simulink model "zcu102_f_200msps_64i_4096c"  (R2021a)
settings: Solver=VariableStepDiscrete  StartTime=0.0  StopTime=10.0  RelTol=1e-3
undefined symbols (not defined in the file or in its folder): datetime, pfb_coeff_gen_calc, posixtime
libraries: xbsIndex_r4 x49646, casper_library_misc x229, casper_library_delays x133, xps_library x20, casper_library_sources x4, xbsReplacements_r4 x4
(part 1 of 502)
settings Model: ModifiedComment=casperlwa -- Wed Dec 18 16:21:52 2024 -- Version 5.0  SimulationMode=rapid-accelerator  Creator=mdexter  LastModifiedBy=casperlwa
settings Model: ModifiedHistory=|
  | casperlwa -- Wed Dec 18 16:21:52 2024 -- Version 5.0
  | 
  | kplant -- Fri Aug 11 09:07:04 2023 -- Version 1.663
  | Saving after compiling
  | 
  | kplant -- Thu Aug 10 22:12:33 2023 -- Version 1.662
  | Version 7.3.2.1 now has pipelines with a latency of 3 before the f-engine's ethernet block.
  | 
  | kplant -- Thu Aug 10 10:59:50 2023 -- Version 1.661
  | Version 7.3.2.0 increases the pipeline latency to 2 in front of the f-engine 40Gbe block and changes the target clock rate to 196 MHz.
  | 
  | kplant -- Wed Aug 09 13:06:15 2023 -- Version 1.660
  | Added pipeline blocks with a latency of 1 before each of the inputs to the 40Gb ethernet block that the f-engine uses.
  | 
  | kplant -- Tue Aug 08 14:00:49 2023 -- Version 1.659
  | 
  | kplant -- Tue Aug 08 13:55:20 2023 -- Version 1.658
  | Adding cosmic ray system version 6.0.1.0 which has the FIR and so far has only been compiled from snap2_f_200msps_64i_4096c_nopfb.slx
  | 
  | kplant -- Thu Apr 27 20:02:10 2023 -- Version 1.657
  | Saving after compile.
  | 
  | kplant -- Wed Apr 26 17:00:51 2023 -- Version 1.656
  | I removed the delay trigger option and changed it to an option to delay the input to the buffer, in order to be able to position the pulse in the center of the buffer.
  | 
  | kplant -- Fri Apr 07 12:31:25 2023 -- Version 1.655
  | Add cosmic ray version 5.0.0.1 and change main version to 7.3.0.2.
  | 
  | kplant -- Thu Apr 06 14:32:58 2023 -- Version 1.654
  | Saving after compiling 7.3.0.1.
  | 
  | jackh -- Thu Apr 06 12:53:56 2023 -- Version 1.653
  | 
  | Make CR block and mask block use sync_time, not telescope_time
  | 
  | kplant -- Fri Mar 24 06:24:14 2023 -- Version 1.652
  | Saving after compiling version 7.3 with cosmic ray system 5.0.0.
  | 
  | jackh -- Wed Mar 08 22:10:30 2023 -- Version 1.651
  | 
  | compiled
  | 
  | jackh -- Wed Mar 08 15:15:31 2023 -- Version 1.650
  | rev 7.2
  | 2 bits below EQ binary point
  | 
  | jackh -- Thu Feb 16 17:13:47 2023 -- Version 1.649
  | 
  | compiled
  | 
  | jackh -- Thu Feb 16 09:43:58 2023 -- Version 1.648
  | 
  | Remove hard-coded first 5-stages of shift
  | 
  | jackh -- Wed Feb 15 17:04:22 2023 -- Version 1.647
  | 
  | compiled
  | 
  | jackh -- Tue Feb 14 18:32:23 2023 -- Version 1.646
  | 
  | Change EQ fractional bits from 5 to 4
  | Rebuild FFT IP with all serial stages optional shift
  | 
  | jackh -- Thu Jan 05 10:26:17 2023 -- Version 1.645
  | v7.0.0 as compiled
  | 
  | jackh -- Wed Jan 04 19:36:26 2023 -- Version 1.644
  | 
  | jackh -- Wed Jan 04 19:34:58 2023 -- Version 1.643
  | v7.0.0.0
  | CR version 4.3.1
  | 
  | jackh -- Tue Dec 20 11:37:43 2022 -- Version 1.642
  | 
  | compiled 6.1. 4 x 0.003ns timing errors, only on unused CR 40GbE RX path.
  | 
  | 
  | jackh -- Fri Dec 16 15:06:04 2022 -- Version 1.641
  | 
  | jackh -- Fri Dec 16 14:46:34 2022 -- Version 1.640
  | 
  | jackh -- Fri Dec 16 14:13:03 2022 -- Version 1.639
  | 
  | jackh -- Fri Dec 16 13:41:06 2022 -- Version 1.638
  | 
  | 6.1 -- add per-signal flag threshold
  | 
  | jackh -- Fri Dec 16 11:47:24 2022 -- Version 1.637
  | 
  | jackh -- Fri Dec 16 11:47:00 2022 -- Version 1.636
  | 
  | jackh -- Thu Dec 15 20:54:27 2022 -- Version 1.635
  | 
  | Add acc_time registers
  | 
  | jackh -- Thu Dec 15 12:49:42 2022 -- Version 1.634
  | 
  | Include all 4 mask cores (whoops!)
  | 
  | jackh -- Thu Dec 15 12:39:59 2022 -- Version 1.633
  | 
  | Change max coarse delay to 4096 (previous compile didn't meet timing)
  | 
  | jackh -- Wed Dec 14 18:27:06 2022 -- Version 1.632
  | 
  | Add "mask" block, which allows zero-ing out spectra
  | whose total power exceed a runtime-controllable
  | threshold
  | 
  | kplant -- Wed Jun 01 13:17:05 2022 -- Version 1.631
  | 
  | kplant -- Wed Jun 01 07:18:09 2022 -- Version 1.630
  | 
  | kplant -- Wed Jun 01 06:41:48 2022 -- Version 1.629
  | Fixed telescope time input to cosmic ray block.
  | 
  | kplant -- Wed Jun 01 06:39:11 2022 -- Version 1.628
  | Commented out scopes
  | 
  | kplant -- Tue May 31 12:58:33 2022 -- Version 1.627
  | Cosmic ray version 4.1. Takes in telescope time, attempts to fix bug where trigger signal is blocked from starting packetizer.
  | 
  | kplant -- Tue May 31 12:56:22 2022 -- Version 1.626
  | 
  | kplant -- Tue May 31 09:44:09 2022 -- Version 1.625
  | 
  | jackh -- Fri May 13 14:05:09 2022 -- Version 1.624
  | 
  | Compiled 5.1.1.0 (CR rev 4)
  | 
  | jackh -- Thu May 12 12:01:29 2022 -- Version 1.623
  | 
  | Add PPS count register (which software already assumes exists!)
  | 
  | jackh -- Thu May 12 11:34:03 2022 -- Version 1.622
  | 
  | v5.1.0
  | Manual merge in of v4.0 CR module
  | 
  | jackh -- Thu Apr 14 04:49:04 2022 -- Version 1.621
  | 
  | jackh -- Wed Mar 23 12:35:09 2022 -- Version 1.620
  | 
  | Manually merce cosmic_ray_system.slx into the cosmic_ray subsystem
  | of this model. cosmic_ray_system appears to be version 3 (based on version register).
  | 
  | Bump main model version to 5.0.0.0
  | 
  | jackh -- Tue Jan 11 12:36:06 2022 -- Version 1.619
  | 
  | jackh -- Mon Jan 10 21:45:35 2022 -- Version 1.618
  | 
  | Bump bugfix
  | 
  | jackh -- Mon Jan 10 21:43:35 2022 -- Version 1.617
  | 
  | jackh -- Mon Jan 10 21:39:42 2022 -- Version 1.616
  | 
  | jackh -- Mon Jan 10 21:33:55 2022 -- Version 1.615
  | 
  | jackh -- Mon Jan 10 21:16:30 2022 -- Version 1.614
  | 
  | Fix bit width of sum(sq(ADC value)) -- was configured for 8 bit ADCs!
  | 
  | jackh -- Mon Nov 15 21:56:57 2021 -- Version 1.613
  | 
  | compiled
  | 
  | jackh -- Mon Nov 15 11:54:45 2021 -- Version 1.612
  | 
  | v4.6.0 -- add internal loopback for sync logic
  | 
  | jackh -- Fri Sep 17 19:23:29 2021 -- Version 1.611
  | 
  | Compiled with clean timing
  | 
  | 
  | jackh -- Fri Sep 17 13:13:59 2021 -- Version 1.610
  | 4.5.4 CR update
  | 
  | jackh -- Tue Sep 14 19:01:48 2021 -- Version 1.609
  | 
  | jackh -- Tue Sep 14 10:37:44 2021 -- Version 1.608
  | 
  | Change CR buffer BRAMs to have latency 2 and optimize for speed.
  | 
  | jackh -- Mon Sep 13 20:49:56 2021 -- Version 1.607
  | 
  | jackh -- Mon Sep 13 20:30:25 2021 -- Version 1.606
  | 
  | 4.5.3
  | Increase latency of autocorr vacc adders to 4
  | Change CR veto pulse extend subtract-1 to implement behaviourally
  | 
  | jackh -- Mon Sep 13 20:29:50 2021 -- Version 1.605
  | 
  | jackh -- Mon Sep 13 10:57:29 2021 -- Version 1.604
  | 
  | v4.5.2
  | 
  | Update CR subsys. Not sure whether this has actually changed,
  | but there was a commit to the repo. Using 14655219acb09ca
  | 
  | jackh -- Mon Sep 13 10:51:07 2021 -- Version 1.603
  | 
  | jackh -- Sun Sep 12 12:08:30 2021 -- Version 1.602
  | 
  | 4.5.1 -- update CR subsys
  | 
  | jackh -- Tue Aug 31 13:12:34 2021 -- Version 1.601
  | 
  | jackh -- Wed Aug 25 19:11:33 2021 -- Version 1.600
  | 
  | jackh -- Tue Aug 24 13:10:24 2021 -- Version 1.599
  | 
  | Fix delay readout bug
  | 
  | jackh -- Tue Aug 24 12:10:39 2021 -- Version 1.598
  | 
  | compiled. Missed timing, but only on 40G RX paths and within the 200MHz->196MHz margin
  | 
  | jackh -- Mon Aug 23 18:54:36 2021 -- Version 1.597
  | 
  | update CR
  | 
  | jackh -- Mon Aug 23 18:52:51 2021 -- Version 1.596
  | 
  | compiled
  | 
  | jackh -- Mon Aug 16 15:01:28 2021 -- Version 1.595
  | 
  | re-fix delay readout
  | 
  | jackh -- Mon Aug 16 14:36:11 2021 -- Version 1.594
  | 
  | compiled v4.3.0
  | 
  | jackh -- Fri Aug 13 12:33:00 2021 -- Version 1.593
  | 
  | jackh -- Fri Aug 13 12:32:21 2021 -- Version 1.592
  | 
  | jackh -- Fri Aug 13 12:30:04 2021 -- Version 1.591
  | 
  | Make autocorr vacc all float32
  | 
  | jackh -- Fri Aug 13 12:16:09 2021 -- Version 1.590
  | 
  | jackh -- Fri Aug 13 12:09:57 2021 -- Version 1.589
  | 
  | jackh -- Thu Aug 12 18:59:45 2021 -- Version 1.588
  | 
  | jackh -- Thu Aug 12 10:15:56 2021 -- Version 1.587
  | 
  | Remove CR 40GbE TX snapshot
  | 
  | jackh -- Thu Aug 12 10:13:47 2021 -- Version 1.586
  | 
  | Misc pipelining
  | 
  | jackh -- Wed Aug 11 12:31:06 2021 -- Version 1.585
  | 
  | jackh -- Wed Aug 11 12:26:16 2021 -- Version 1.584
  | 
  | v4.4
  | 
  | jackh -- Wed Aug 11 12:25:20 2021 -- Version 1.583
  | 
  | Combine some software registers in CR subsys
  | Combine delay registers into a RAM to (try to) reduce wishbone interconnect
  | 
  | jackh -- Wed Aug 11 11:56:18 2021 -- Version 1.582
  | 
  | Change delay registering to store delays in bram
  | 
  | jackh -- Wed Aug 11 11:10:53 2021 -- Version 1.581
  | 
  | Reduce number of noise generators to 4.
  | Control noise fanout to multiplexers
  | 
  | jackh -- Tue Aug 10 11:27:32 2021 -- Version 1.580
  | 
  | jackh -- Tue Aug 10 11:26:01 2021 -- Version 1.579
  | 
  | fix EQ coefficient addressing
  | 
  | jackh -- Tue Aug 10 11:02:45 2021 -- Version 1.578
  | 
  | jackh -- Mon Aug 09 21:32:19 2021 -- Version 1.577
  | 
  | jackh -- Mon Aug 09 21:31:35 2021 -- Version 1.576
  | 
  | jackh -- Mon Aug 09 18:30:08 2021 -- Version 1.575
  | 
  | compiled
  | 
  | jackh -- Mon Aug 09 10:43:25 2021 -- Version 1.574
  | 
  | jackh -- Mon Aug 09 10:40:58 2021 -- Version 1.573
  | 
  | jackh -- Mon Aug 09 10:31:43 2021 -- Version 1.572
  | 
  | Add pipeline registers on high-fanout CR subsystem sw register outputs
  | 
  | jackh -- Sun Aug 08 14:34:28 2021 -- Version 1.571
  | 
  | v4.3.0
  | 
  | jackh -- Sun Aug 08 14:24:45 2021 -- Version 1.570
  | 
  | jackh -- Sun Aug 08 14:11:25 2021 -- Version 1.569
  | 
  | jackh -- Sun Aug 08 13:31:26 2021 -- Version 1.568
  | 
  | Go back to floating point autocorr. Previous design used more ram and failed timing
  | 
  | jackh -- Sat Aug 07 15:01:43 2021 -- Version 1.567
  | 
  | Change chan_reorder double buffers to optimize for area
  | (sometimes halves the number of brams)
  | 
  | jackh -- Sat Aug 07 14:54:24 2021 -- Version 1.566
  | 
  | v4.2.0
  | 
  | Make autocorr 64-bit fixed point (to save floating cast)
  | Remove serial reorder from FFT and make part of EQ.
  | Compensate by bitbashing addresses for autocorr readout and EQ coefficients
  | 
  | 
  | jackh -- Sat Aug 07 14:49:26 2021 -- Version 1.565
  | 
  | jackh -- Sat Aug 07 14:41:23 2021 -- Version 1.564
  | 
  | jackh -- Sat Aug 07 14:24:43 2021 -- Version 1.563
  | 
  | jackh -- Sat Aug 07 13:38:58 2021 -- Version 1.562
  | 
  | Try to optimize pre-PFB reorder buffers
  | 
  | jackh -- Sat Aug 07 13:18:16 2021 -- Version 1.561
  | 
  | jackh -- Sat Aug 07 13:16:02 2021 -- Version 1.560
  | 
  | Put back in clip count registers because LRD uses them
  | 
  | jackh -- Sat Aug 07 12:39:11 2021 -- Version 1.559
  | 
  | jackh -- Sat Aug 07 12:19:25 2021 -- Version 1.558
  | 
  | compiled. Missed timing on one path by 0.04ns
  | 
  | jackh -- Tue Aug 03 11:43:54 2021 -- Version 1.557
  | 
  | jackh -- Tue Aug 03 11:14:29 2021 -- Version 1.556
  | 
  | Fix double up of delay RAM
  | 
  | jackh -- Tue Aug 03 11:04:58 2021 -- Version 1.555
  | 
  | 4.2.1 -- put CR QSP on port 2
  | 
  | jackh -- Mon Aug 02 12:46:12 2021 -- Version 1.554
  | 
  | Remove RX-side debug counters in CR 40G core
  | 
  | jackh -- Mon Aug 02 12:36:50 2021 -- Version 1.553
  | 
  | Make input power calculation use DSP
  | Change some input delays to behavioural
  | Change delay block to implement a single delay
  | with programmable delay from 0 to 1023, followed by 7
  | fixed 1024 clock delays which may be optionally bypassed.
  | Hopefully this will use less power.
  | 
  | jackh -- Mon Aug 02 11:15:00 2021 -- Version 1.552
  | 
  | implementation optimizations in CR block
  | 
  | jackh -- Mon Aug 02 10:53:02 2021 -- Version 1.551
  | 
  | jackh -- Sun Aug 01 12:01:07 2021 -- Version 1.550
  | make CR use LED 3 to prevent clash
  | 
  | jackh -- Sun Aug 01 10:17:04 2021 -- Version 1.549
  | 
  | v 4.1.0
  | uncomment CR.
  | 
  | jackh -- Sun Aug 01 10:02:19 2021 -- Version 1.548
  | 
  | jackh -- Sat Jul 31 15:41:04 2021 -- Version 1.547
  | v4.0.4 -- prep to compile with pre-placement power opt
  | 
  | jackh -- Sat Jul 31 11:19:09 2021 -- Version 1.546
  | 
  | jackh -- Fri Jul 30 17:49:37 2021 -- Version 1.545
  | 
  | jackh -- Fri Jul 30 17:45:17 2021 -- Version 1.544
  | 
  | rev 4.0.3
  | 
  | jackh -- Fri Jul 30 17:36:17 2021 -- Version 1.543
  | 
  | make autocorr float cast latency 4 again (failed timing)
  | set all map latencies on pre-pfb reorder to 2 (failed timing)
  | 
  | Copy all pfb cores so they match. A bug was introduced where core 0
  | had a 1 cycle latency difference to the others
  | 
  | jackh -- Fri Jul 30 12:38:28 2021 -- Version 1.542
  | 
  | shared bram latency to 1 in corr block
  | 
  | jackh -- Fri Jul 30 12:37:15 2021 -- Version 1.541
  | 
  | reduce shared bram latency to 1 in eqtvg
  | 
  | jackh -- Fri Jul 30 12:35:00 2021 -- Version 1.540
  | 
  | Reduce shared bram latency to 1 in autocorr and EQ blocks
  | 
  | jackh -- Fri Jul 30 12:31:43 2021 -- Version 1.539
  | 
  | Add option to tie autocorr data inputs to zero (to save a little power)
  | Reduce autocorr mux input latency 2->1
  | Reduce autocorr floating point cast latency 4->3
  | 
  | jackh -- Fri Jul 30 11:48:33 2021 -- Version 1.538
  | 
  | 4.0.2
  | Optimize bram delays for power
  | 
  | jackh -- Fri Jul 30 11:45:47 2021 -- Version 1.537
  | 
  | compiled
  | 
  | jackh -- Thu Jul 29 17:56:15 2021 -- Version 1.536
  | 
  | 4.0.1
  | 
  | Make a couple of brams optimize for power. Rebuild FFT / FIR to use (hopefully) less logic.
  | 
  | jackh -- Tue Jul 27 18:46:01 2021 -- Version 1.535
  | 
  | jackh -- Tue Jul 27 16:53:09 2021 -- Version 1.534
  | 
  | add in CR firmware (but comment out)
  | bump version to 4.0.0
  | 
  | 
  | jackh -- Tue Jul 27 16:23:54 2021 -- Version 1.533
  | 
  | jackh -- Tue Jul 27 16:22:45 2021 -- Version 1.532
  | 
  | Make 4-bit quantize use DSP core. Remove 4-bit clip count. it's basically useless unless broken down by freq channel
  | 
  | jackh -- Tue Jul 27 16:11:15 2021 -- Version 1.531
  | 
  | jackh -- Tue Jul 27 10:57:43 2021 -- Version 1.530
  | 
  | as compiled
  | 
  | jackh -- Mon Jul 26 20:52:28 2021 -- Version 1.529
  | 
  | jackh -- Mon Jul 26 20:39:42 2021 -- Version 1.528
  | 
  | jackh -- Mon Jul 26 14:40:36 2021 -- Version 1.527
  | 
  | Add IODELAYs on timing signals. Add ext PPS variations register
  | 
  | 
  | jackh -- Mon Jul 26 13:07:19 2021 -- Version 1.526
  | 
  | jackh -- Mon Jul 26 13:02:55 2021 -- Version 1.525
  | 
  | jackh -- Mon Jul 26 12:04:41 2021 -- Version 1.524
  | 
  | jackh -- Fri Jul 23 16:52:58 2021 -- Version 1.523
  | 
  | jackh -- Fri Jul 23 16:41:18 2021 -- Version 1.522
  | 
  | jackh -- Thu Jul 22 17:52:28 2021 -- Version 1.521
  | 
  | jackh -- Thu Jul 22 12:41:40 2021 -- Version 1.520
  | 
  | jackh -- Thu Jul 22 12:33:28 2021 -- Version 1.519
  | 
  | jackh -- Thu Jul 22 12:03:06 2021 -- Version 1.518
  | 
  | jackh -- Thu Jul 22 12:02:39 2021 -- Version 1.517
  | 
  | jackh -- Thu Jul 22 11:51:17 2021 -- Version 1.516
  | 
  | rev 3.4
  | 
  | jackh -- Thu Jul 22 11:33:52 2021 -- Version 1.515
  | 
  | Add trigger logic to autocorr spectrum grabbing
  | 
  | jackh -- Wed Jul 14 19:19:47 2021 -- Version 1.514
  | 
  | Bump version to 3.3.0
  | 
  | jackh -- Wed Jul 14 19:18:36 2021 -- Version 1.513
  | 
  | Add PFB FIR bypass
  | 
  | 
  | jackh -- Tue Jul 06 15:20:17 2021 -- Version 1.512
  | 
  | jackh -- Thu Jul 01 15:44:46 2021 -- Version 1.511
  | 
  | jackh -- Thu Jul 01 15:41:36 2021 -- Version 1.510
  | 
  | jackh -- Thu Jul 01 15:38:13 2021 -- Version 1.509
  | 
  | Bump version to 3.2.1
  | 
  | jackh -- Thu Jul 01 14:31:27 2021 -- Version 1.508
  | 
  | Add enable to ADC external chip sync
  | 
  | jackh -- Thu Jul 01 12:43:11 2021 -- Version 1.507
  | as compiled
  | 
  | jackh -- Wed Jun 30 19:07:36 2021 -- Version 1.506
  | 
  | jackh -- Wed Jun 30 19:06:42 2021 -- Version 1.505
  | 
  | Bugfix 1 -- double-buffered reorders seem broken?!
  | 
  | jackh -- Tue Jun 29 18:31:06 2021 -- Version 1.504
  | 
  | jackh -- Tue Jun 29 18:30:15 2021 -- Version 1.503
  | 
  | jackh -- Tue Jun 29 14:35:01 2021 -- Version 1.502
  | 
  | Add PPS period counter.
  | Extend output sync pulse to 1024 clocks
  | 
  | jackh -- Thu Jun 17 11:51:33 2021 -- Version 1.501
  | 
  | jackh -- Wed Jun 16 11:07:37 2021 -- Version 1.500
  | 
  | jackh -- Wed Jun 16 11:02:07 2021 -- Version 1.499
  | 
  | Bump version to 3.1.0
  | 
  | Add Zynq interfaces:
  |   - Power monitoring I2C
  |   - PPS GPIO input
  | 
  | Requires Zynq to be programmed with appropriate bitstream.
  | 
  | jackh -- Tue Jun 15 15:37:49 2021 -- Version 1.498
  | 
  | Change ADC versions to rev 2 (updated pinout)
  | Bump firmware version to 3.0.0
  | 
  | jackh -- Thu Jun 10 13:30:51 2021 -- Version 1.497
  | 
  | bugfix 3
  | 
  | Off-by-1 autocorr channel error
  | 
  | jackh -- Thu Jun 10 12:13:26 2021 -- Version 1.496
  | 
  | compile. Bump bugfix to 2
  | 
  | jackh -- Wed Jun 09 12:36:01 2021 -- Version 1.495
  | 
  | FFT of bit inversion fix
  | 
  | jackh -- Tue Jun 08 19:39:36 2021 -- Version 1.494
  | 
  | jackh -- Tue Jun 08 18:24:07 2021 -- Version 1.493
  | 
  | off-by-one integration bug in autocorr acc
  | 
  | jackh -- Fri May 14 17:02:59 2021 -- Version 1.492
  | 
  | Remove a pipeline register after the ADCs.
  | Trying to free up routing at the FIFO inputs to make the compiler's life easier.
  | 
  | jackh -- Fri May 14 09:09:53 2021 -- Version 1.491
  | 
  | as compiled
  | 
  | jackh -- Wed May 05 14:45:26 2021 -- Version 1.490
  | 
  | Version bump prior to recompiling with new ADC interface (with bitslip)
  | 
  | jackh -- Tue Apr 13 20:04:05 2021 -- Version 1.489
  | 
  | jackh -- Tue Apr 13 20:01:34 2021 -- Version 1.488
  | 
  | docstrings
  | 
  | jackh -- Tue Apr 13 19:38:13 2021 -- Version 1.487
  | 
  | updates OK
  | 
  | jackh -- Tue Apr 13 19:29:45 2021 -- Version 1.486
  | 
  | docstrings
  | 
  | jackh -- Tue Apr 13 19:14:40 2021 -- Version 1.485
  | 
  | docstrings in sync subsys
  | 
  | jackh -- Tue Apr 13 19:00:08 2021 -- Version 1.484
  | 
  | jackh -- Tue Apr 13 18:57:55 2021 -- Version 1.483
  | 
  | update 40G core with docstrings
  | 
  | 
  | jackh -- Tue Apr 13 18:42:31 2021 -- Version 1.482
  | 
  | jackh -- Tue Apr 13 18:20:50 2021 -- Version 1.481
  | 
  | docstrings
  | 
  | jackh -- Tue Apr 13 17:57:44 2021 -- Version 1.480
  | 
  | jackh -- Tue Apr 13 17:52:27 2021 -- Version 1.479
  | 
  | jackh -- Tue Apr 13 17:44:36 2021 -- Version 1.478
  | 
  | docstrings
  | 
  | jackh -- Tue Apr 13 17:36:01 2021 -- Version 1.477
  | 
  | docstrings
  | 
  | jackh -- Tue Apr 13 17:25:32 2021 -- Version 1.476
  | 
  | jackh -- Tue Apr 13 17:20:03 2021 -- Version 1.475
  | 
  | jackh -- Tue Apr 13 17:12:39 2021 -- Version 1.474
  | 
  | regenerate brams including autodocs
  | 
  | jackh -- Tue Apr 13 16:48:19 2021 -- Version 1.473
  | 
  | update software registers to include docstrings
  | 
  | jackh -- Tue Apr 13 16:04:09 2021 -- Version 1.472
  | 
  | jackh -- Tue Apr 13 10:32:10 2021 -- Version 1.471
  | 
  | Update software registers to include description field.
  | Step 1 in the grand plan to autodocument
  | 
  | jackh -- Thu Apr 08 11:57:43 2021 -- Version 1.470
  | 
  | Change FFT overflow registers to counters rather than flags
  | 
  | jackh -- Sun Mar 28 18:37:18 2021 -- Version 1.469
  | 
  | Misc timing internals pipelining
  | 
  | jackh -- Sun Mar 28 18:35:09 2021 -- Version 1.468
  | 
  | Unsurprisingly, a 64-bit DSP counter isn't allowed :)
  | Use HDL
  | 
  | jackh -- Sun Mar 28 18:29:55 2021 -- Version 1.467
  | 
  | Implement TT counter with DSP
  | 
  | jackh -- Sun Mar 28 18:28:20 2021 -- Version 1.466
  | 
  | rev up to 2.2.2
  | 
  | jackh -- Sun Mar 28 18:27:31 2021 -- Version 1.465
  | 
  | Add pipelining to sync_in GPIO
  | 
  | jackh -- Sun Mar 28 17:31:26 2021 -- Version 1.464
  | 
  | bump version to 2.2.1
  | 
  | jackh -- Sun Mar 28 17:20:11 2021 -- Version 1.463
  | 
  | jackh -- Sun Mar 28 17:17:29 2021 -- Version 1.462
  | 
  | Fix to spectra count latency in autocorrelation
  | 
  | jackh -- Sun Mar 28 16:47:41 2021 -- Version 1.461
  | 
  | Reduce max acc_len to 17 bits to match control register
  | 
  | jackh -- Sun Mar 28 16:04:04 2021 -- Version 1.460
  | 
  | jackh -- Sun Mar 28 10:30:48 2021 -- Version 1.459
  | 
  | 
  | 
  | jackh -- Sat Mar 27 16:35:07 2021 -- Version 1.458
  | 
  | jackh -- Sat Mar 27 16:33:52 2021 -- Version 1.457
  | 
  | jackh -- Sat Mar 27 16:33:04 2021 -- Version 1.456
  | 
  | Mux autocorrs to free up RAM. Prep to rebuild with interleaved PFB, which fell out the library
  | 
  | jackh -- Sat Mar 27 14:15:08 2021 -- Version 1.455
  | 
  | inconsequential block name changes in noise generator
  | 
  | jackh -- Sat Mar 27 14:01:25 2021 -- Version 1.454
  | 
  | remove posedges from ADC control signals
  | (some of these need to be registered into a domain with a clock half the rate of simulink)
  | 
  | jackh -- Fri Mar 19 15:12:44 2021 -- Version 1.453
  | 
  | jackh -- Tue Mar 16 12:34:32 2021 -- Version 1.452
  | 
  | Add internal ADC sync register. Bump version to 2.1.0
  | 
  | jackh -- Tue Mar 16 11:03:32 2021 -- Version 1.451
  | 
  | jackh -- Tue Mar 09 10:10:05 2021 -- Version 1.450
  | 
  | jackh -- Tue Mar 09 10:05:23 2021 -- Version 1.449
  | 
  | jackh -- Tue Mar 09 10:03:37 2021 -- Version 1.448
  | 
  | jackh -- Mon Mar 01 11:44:07 2021 -- Version 1.447
  | 
  | jackh -- Sun Feb 28 13:35:39 2021 -- Version 1.446
  | 
  | update clocks to use FMC1 (since that's the only prototype ADC board we can use)
  | 
  | jackh -- Sun Feb 28 13:26:05 2021 -- Version 1.445
  | 
  | Pipelining on ADC output; 40GbE input
  | 
  | jackh -- Sat Feb 27 12:53:30 2021 -- Version 1.444
  | 
  | jackh -- Fri Feb 26 19:07:36 2021 -- Version 1.443
  | 
  | jackh -- Fri Feb 26 19:03:07 2021 -- Version 1.442
  | 
  | Store autocorrs as 32-bit floats (to save RAM)
  | 
  | jackh -- Thu Feb 25 20:40:57 2021 -- Version 1.441
  | 
  | jackh -- Thu Feb 25 20:35:24 2021 -- Version 1.440
  | 
  | autocorr fixes
  | 
  | 
  | jackh -- Tue Feb 23 18:00:30 2021 -- Version 1.439
  | 
  | Add snapshot trigger to ADC(s)
  | 
  | jackh -- Tue Feb 23 16:55:19 2021 -- Version 1.438
  | 
  | jackh -- Tue Feb 23 16:44:18 2021 -- Version 1.437
  | 
  | Add unquantized autocorr module
  | 
  | jackh -- Tue Feb 02 19:39:04 2021 -- Version 1.436
  | 
  | add adc_sync output
  | 
  | jackh -- Mon Dec 07 12:03:10 2020 -- Version 1.435
  | 
  | update ADCs to rev2
  | 
  | jackh -- Thu Nov 19 12:32:34 2020 -- Version 1.434
  | 
  | Updates to 4-bit quantize for timing improvements (hopefully)
  | 
  | jackh -- Tue Nov 17 11:51:00 2020 -- Version 1.433
  | 
  | Add rev / bugfix fields to version.
  | Add timestamp register -- storing UNIX timestamp of build (or, strictly, of last update time, I think)
  | 
  | 
  | jackh -- Tue Aug 11 17:16:40 2020 -- Version 1.432
  | 
  | snap2 -> rev 2
  | 
  | jackh -- Tue Aug 11 17:13:54 2020 -- Version 1.431
  | 
  | compiled
  | 
  | jackh -- Tue Aug 11 10:34:36 2020 -- Version 1.430
  | 
  | sync subsystem cleanup.
  | Add sync_time to packet header
  | 
  | jackh -- Tue Jul 28 17:40:14 2020 -- Version 1.429
  | 
  | Make some blocks  with registers inside gold
  | 
  | jackh -- Tue Jun 16 16:41:05 2020 -- Version 1.428
  | 
  | jackh -- Mon Jun 15 10:48:06 2020 -- Version 1.427
  | 
  | jackh -- Fri Jun 12 12:44:37 2020 -- Version 1.426
  | 
  | add chan_block_index field to output headers.
  | 
  | This should allow compatibility with the bifrost UDP capture framework
  | 
  | jackh -- Thu Jun 11 12:01:09 2020 -- Version 1.425
  | 
  | Update SNAP2 MSSGE block (new version has choice of FMC0/1 clock sourcing and SNAP2 hardware version selection)
  | 
  | jackh -- Thu Jun 11 11:59:20 2020 -- Version 1.424
  | update ADC blocks for single-FMC versions. Add ADC sync and reset registers
  | 
  | jackh -- Thu Jun 11 11:55:51 2020 -- Version 1.423
  | 
  | jackh -- Thu Jun 11 11:54:30 2020 -- Version 1.422
  | 
  | default n_pols to 64
  | 
  | jackh -- Thu Jun 11 11:44:03 2020 -- Version 1.421
  | 
  | make dest port 16-bits
  | add n_pols header entry
  | 
  | jackh -- Thu Jun 11 11:17:26 2020 -- Version 1.420
  | 
  | extra reserved 32-bit header entry
  | 
  | jackh -- Thu Jun 11 11:14:09 2020 -- Version 1.419
  | 
  | Reorg packet header to include n_chans, magic number, and reserver 32-bits
  | 
  | jackh -- Sun May 10 22:08:41 2020 -- Version 1.418
  | 
  | Make destination port selection per-packet
  | 
  | jackh -- Sun May 10 19:32:52 2020 -- Version 1.417
  | 
  | update chan-reorder to reorder pols in ascending order
  | 
  | jackh -- Fri May 08 15:11:27 2020 -- Version 1.416
  | 
  | update input block with RMS timing fixes
  | 
  | jackh -- Fri May 08 15:10:21 2020 -- Version 1.415
  | 
  | jackh -- Tue May 05 13:29:10 2020 -- Version 1.414
  | 
  | jackh -- Fri May 01 15:26:24 2020 -- Version 1.413
  | 
  | Use sys_clk for testing
  | 
  | jackh -- Fri May 01 15:14:39 2020 -- Version 1.412
  | 
  | Rename 1Gbe core so it is alphabetically first
  | 
  | jackh -- Fri May 01 15:11:49 2020 -- Version 1.411
  | 
  | jackh -- Fri May 01 11:15:12 2020 -- Version 1.410
  | 
  | Change 40gbe core enable so that software enable state can only change immediately after an EOF
  | 
  | jackh -- Fri Apr 24 17:52:32 2020 -- Version 1.409
  | 
  | jackh -- Fri Apr 24 17:51:51 2020 -- Version 1.408
  | 
  | jackh -- Fri Apr 24 17:50:48 2020 -- Version 1.407
  | 
  | jackh -- Fri Apr 24 17:48:49 2020 -- Version 1.406
  | 
  | make packetizer simpler
  | 
  | jackh -- Fri Apr 24 16:17:31 2020 -- Version 1.405
  | 
  | ass max_delay register to delay core
  | 
  | jackh -- Fri Apr 24 16:14:09 2020 -- Version 1.404
  | 
  | jackh -- Fri Apr 24 16:12:03 2020 -- Version 1.403
  | 
  | update eq_tvg to single bram
  | 
  | jackh -- Fri Apr 24 16:08:40 2020 -- Version 1.402
  | 
  | jackh -- Thu Apr 09 09:24:59 2020 -- Version 1.401
  | 
  | make delays 8k long
  | 
  | jackh -- Mon Mar 30 21:41:54 2020 -- Version 1.400
  | 
  | add 1gbe core
  | 
  | jackh -- Sat Mar 14 14:05:43 2020 -- Version 1.399
  | 
  | jackh -- Wed Feb 19 18:48:51 2020 -- Version 1.398
  | 
  | jackh -- Wed Feb 19 18:31:56 2020 -- Version 1.397
  | 
  | jackh -- Wed Feb 19 18:25:06 2020 -- Version 1.396
  | 
  | jackh -- Wed Feb 19 17:10:23 2020 -- Version 1.395
  | 
  | add back in reorders in pfb (having fixed mlib_devel)
  | 
  | jackh -- Tue Feb 18 17:39:49 2020 -- Version 1.394
  | 
  | 10 bitify
  | 
  | jackh -- Tue Feb 18 17:31:23 2020 -- Version 1.393
  | 
  | update_casper_blocks
  | 
  | jackh -- Fri Jan 24 08:04:16 2020 -- Version 1.392
  | replace ADC with sw regs to try and compile for SNAP2
  | 
  | jackh -- Wed Jan 08 08:41:40 2020 -- Version 1.391
  | 
  | 
  | jackh -- Wed Jan 08 08:26:20 2020 -- Version 1.390
  | 
  | 
  | jackh -- Wed Jan 08 08:24:50 2020 -- Version 1.389
  | 
  | 
  | jackh -- Wed Jan 08 08:23:40 2020 -- Version 1.388
  | 
  | 
  | jackh -- Wed Jan 08 08:22:29 2020 -- Version 1.387
  | update platform to SNAP2
  | 
  | jackh -- Wed Jan 08 08:21:36 2020 -- Version 1.386
  | 
  | 
  | jackh -- Wed Jan 08 08:21:09 2020 -- Version 1.385
  | Update internal cross-corr block
  | 
  | jackh -- Wed Jan 08 07:50:59 2020 -- Version 1.384
  | 
  | 
  | jackh -- Wed Jan 08 07:48:46 2020 -- Version 1.383
  | 
  | 
  | jackh -- Wed Jan 08 07:45:47 2020 -- Version 1.382
  | 
  | 
  | jackh -- Wed Jan 08 07:44:03 2020 -- Version 1.381
  | 
  | 
  | jackh -- Wed Jan 08 07:41:17 2020 -- Version 1.380
  | 
  | 
  | jackh -- Wed Jan 08 07:35:58 2020 -- Version 1.379
  | 
  | 
  | jackh -- Wed Jan 08 07:33:51 2020 -- Version 1.378
  | 
  | 
  | jackh -- Wed Jan 08 07:29:30 2020 -- Version 1.377
  | 
  | 
  | jackh -- Wed Jan 08 07:24:27 2020 -- Version 1.376
  | 1st pass fuilly updated to LWA spec
  | 
  | jackh -- Tue Jan 07 10:50:49 2020 -- Version 1.375
  | 
  | 
  | jackh -- Tue Jan 07 10:38:05 2020 -- Version 1.374
  | EQ tvg & FFT updated for 64 inputs
  | 
  | jackh -- Tue Jan 07 10:18:18 2020 -- Version 1.373
  | 
  | 
  | jackh -- Tue Jan 07 10:12:36 2020 -- Version 1.372
  | update FFT
  | 
  | jackh -- Tue Jan 07 09:22:52 2020 -- Version 1.371
  | update FFT core (currently only doing 1/4 of the necessary work)
  | 
  | jackh -- Tue Jan 07 07:40:39 2020 -- Version 1.370